simulink library "MOST_Lib"  (R2020b)
settings: Solver=VariableStepAuto  StartTime=0.0  StopTime=10.0  RelTol=1e-3
undefined symbols (not defined in the file or in its folder): simu, wind, windTurbine, windturbine, X, Y, Z
libraries: sm_lib x34, nesl_utility x28, $bdroot x6, simulink_extras x5, simulink x4, WECSim_Lib_Body_Elements x1
(part 2 of 2)

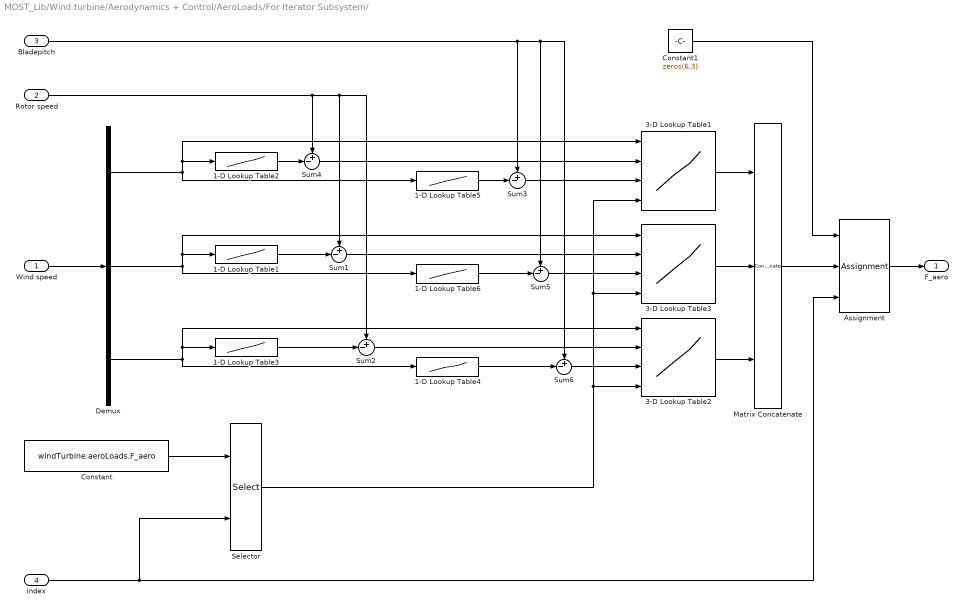
== system MOST_Lib/Wind turbine/Aerodynamics + Control/AeroLoads/For Iterator Subsystem/  ==
  Wind speed : Inport
  Rotor speed : Inport  Port=2
  Bladepitch : Inport  Port=3
  Index : Inport  Port=4
  1-D Lookup Table1 : Lookup_n-D  NumberOfTableDimensions=1  BreakpointsForDimension1=windTurbine.aeroLoads.SS.WINDSPEED  Table=windTurbine.aeroLoads.SS.ROTSPD  InternalRulePriority=Speed  RndMeth=Simplest  InputSameDT=on
  1-D Lookup Table2 : Lookup_n-D  NumberOfTableDimensions=1  BreakpointsForDimension1=windTurbine.aeroLoads.SS.WINDSPEED  Table=windTurbine.aeroLoads.SS.ROTSPD  InternalRulePriority=Speed  RndMeth=Simplest  InputSameDT=on
  1-D Lookup Table3 : Lookup_n-D  NumberOfTableDimensions=1  BreakpointsForDimension1=windTurbine.aeroLoads.SS.WINDSPEED  Table=windTurbine.aeroLoads.SS.ROTSPD  InternalRulePriority=Speed  RndMeth=Simplest  InputSameDT=on
  1-D Lookup Table4 : Lookup_n-D  NumberOfTableDimensions=1  BreakpointsForDimension1=windTurbine.aeroLoads.SS.WINDSPEED  Table=windTurbine.aeroLoads.SS.BLADEPITCH  InternalRulePriority=Speed  RndMeth=Simplest  InputSameDT=on
  1-D Lookup Table5 : Lookup_n-D  NumberOfTableDimensions=1  BreakpointsForDimension1=windTurbine.aeroLoads.SS.WINDSPEED  Table=windTurbine.aeroLoads.SS.BLADEPITCH  InternalRulePriority=Speed  RndMeth=Simplest  InputSameDT=on
  1-D Lookup Table6 : Lookup_n-D  NumberOfTableDimensions=1  BreakpointsForDimension1=windTurbine.aeroLoads.SS.WINDSPEED  Table=windTurbine.aeroLoads.SS.BLADEPITCH  InternalRulePriority=Speed  RndMeth=Simplest  InputSameDT=on
  3-D Lookup Table1 : Lookup_n-D  NumberOfTableDimensions=3  BreakpointsForDimension1=windTurbine.aeroLoads.V  BreakpointsForDimension2=windTurbine.aeroLoads.omegaerr  BreakpointsForDimension3=windTurbine.aeroLoads.thetaerr  TableSource=Input port  Table=squeeze(windturbine.aeroloads.F_aero(:,:,:,1))  InternalRulePriority=Speed  RndMeth=Simplest  InputSameDT=on
      settings: InputPortMap=u0,u1,u2,p221
  3-D Lookup Table2 : Lookup_n-D  NumberOfTableDimensions=3  BreakpointsForDimension1=windTurbine.aeroLoads.V  BreakpointsForDimension2=windTurbine.aeroLoads.omegaerr  BreakpointsForDimension3=windTurbine.aeroLoads.thetaerr  TableSource=Input port  Table=squeeze(windturbine.aeroloads.F_aero(:,:,:,1))  InternalRulePriority=Speed  RndMeth=Simplest  InputSameDT=on
      settings: InputPortMap=u0,u1,u2,p221
  3-D Lookup Table3 : Lookup_n-D  NumberOfTableDimensions=3  BreakpointsForDimension1=windTurbine.aeroLoads.V  BreakpointsForDimension2=windTurbine.aeroLoads.omegaerr  BreakpointsForDimension3=windTurbine.aeroLoads.thetaerr  TableSource=Input port  Table=squeeze(windturbine.aeroloads.F_aero(:,:,:,1))  InternalRulePriority=Speed  RndMeth=Simplest  InputSameDT=on
      settings: InputPortMap=u0,u1,u2,p221
  Assignment : Assignment  NumberOfDimensions=2  DiagnosticForDimensions=Warning  IndexOptions=Index vector (port),Assign all  Indices=1,1  OutputSizes=1,1
  Constant : Constant  Value=windTurbine.aeroLoads.F_aero
  Constant1 : Constant  Value=zeros(6,3)
  Demux : Demux  Outputs=3
  Matrix Concatenate : Concatenate  NumInputs=3  Mode=Multidimensional array  ConcatenateDimension=2
  Selector : Selector  NumberOfDimensions=4  InputPortWidth=3  IndexOptions=Select all,Select all,Select all,Index vector (port)  Indices=[1 3],1,1,1  OutputSizes=1,1,1,1
  Sum{1..6} (6 alike) : Sum  Inputs=+-|
  F_aero : Outport  PortDimensions=[6 3]
  wires:
  Wind speed/1 -> Demux/1
  Demux/3 -> 3-D Lookup Table2/1, 1-D Lookup Table3/1, 1-D Lookup Table4/1
  Demux/2 -> 1-D Lookup Table1/1, 3-D Lookup Table3/1, 1-D Lookup Table6/1
  1-D Lookup Table6/1 -> Sum5/2
  1-D Lookup Table5/1 -> Sum3/2
  1-D Lookup Table4/1 -> Sum6/2
  Sum6/1 -> 3-D Lookup Table2/3
  Sum4/1 -> 3-D Lookup Table1/2
  Sum3/1 -> 3-D Lookup Table1/3
  Demux/1 -> 1-D Lookup Table2/1, 3-D Lookup Table1/1, 1-D Lookup Table5/1
  Sum5/1 -> 3-D Lookup Table3/3
  Sum1/1 -> 3-D Lookup Table3/2
  Sum2/1 -> 3-D Lookup Table2/2
  Rotor speed/1 -> Sum4/1, Sum2/1, Sum1/1
  Constant/1 -> Selector/1
  Index/1 -> Selector/2, Assignment/3
  Selector/1 -> 3-D Lookup Table2/4, 3-D Lookup Table3/4, 3-D Lookup Table1/4
  1-D Lookup Table2/1 -> Sum4/2
  1-D Lookup Table1/1 -> Sum1/2
  1-D Lookup Table3/1 -> Sum2/2
  Bladepitch/1 -> Sum3/1, Sum6/1, Sum5/1
  Assignment/1 -> F_aero/1
  Constant1/1 -> Assignment/1
  3-D Lookup Table1/1 -> Matrix Concatenate/1
  3-D Lookup Table3/1 -> Matrix Concatenate/2
  3-D Lookup Table2/1 -> Matrix Concatenate/3
  Matrix Concatenate/1 -> Assignment/2

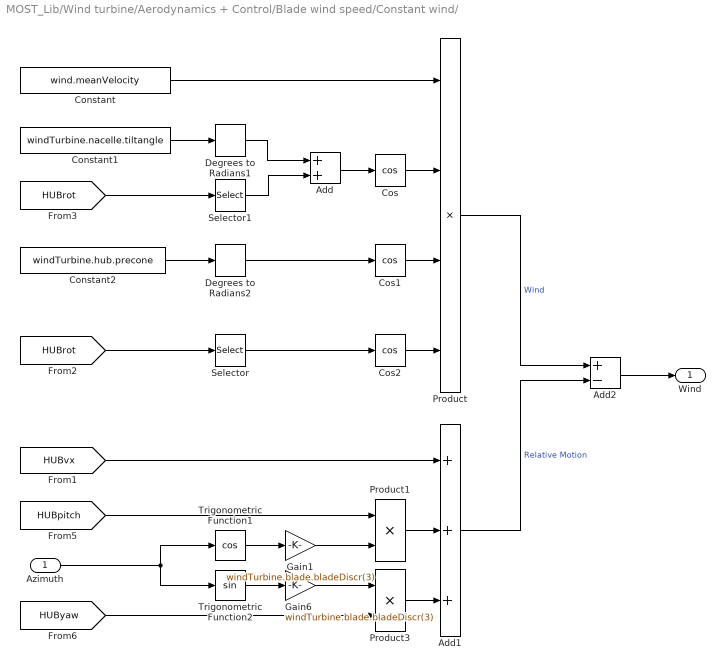
== system MOST_Lib/Wind turbine/Aerodynamics + Control/Blade wind speed/Constant wind/  ==
  Azimuth : Inport
  Add : Sum
  Add1 : Sum  Inputs=+++
  Add2 : Sum  Inputs=+-
  Constant : Constant  Value=wind.meanVelocity
  Constant1 : Constant  Value=windTurbine.nacelle.tiltangle
  Constant2 : Constant  Value=windTurbine.hub.precone
  Cos : Trigonometry  Operator=cos
  Cos1 : Trigonometry  Operator=cos
  Cos2 : Trigonometry  Operator=cos
  Degrees to Radians1 : simulink_extras/Transformations/Degrees to Radians
  Degrees to Radians2 : simulink_extras/Transformations/Degrees to Radians
  Gain1 : Gain  Gain=windTurbine.blade.bladeDiscr(3)
  Gain6 : Gain  Gain=windTurbine.blade.bladeDiscr(3)
  Product : Product  Inputs=4
  Product1 : Product
  Product3 : Product
  Selector : Selector  InputPortWidth=3  IndexOptions=Index vector (dialog)  Indices=[3]  OutputSizes=1
  Selector1 : Selector  InputPortWidth=3  IndexOptions=Index vector (dialog)  Indices=[2]  OutputSizes=1
  Trigonometric Function1 : Trigonometry  Operator=cos
  Trigonometric Function2 : Trigonometry
  Wind : Outport
  wires:
  Cos1/1 -> Product/3
  Selector/1 -> Cos2/1
  Degrees to Radians2/1 -> Cos1/1
  Add/1 -> Cos/1
  Selector1/1 -> Add/2
  Constant1/1 -> Degrees to Radians1/1
  Degrees to Radians1/1 -> Add/1
  Cos2/1 -> Product/4
  Constant2/1 -> Degrees to Radians2/1
  Cos/1 -> Product/2
  Product/1 -> Add2/1  "Wind"
  Constant/1 -> Product/1
  Product3/1 -> Add1/3
  Trigonometric Function2/1 -> Gain6/1
  Gain6/1 -> Product3/1
  Add1/1 -> Add2/2  "Relative Motion"
  [HUBvx] -> Add1/1
  Gain1/1 -> Product1/2
  Product1/1 -> Add1/2
  Azimuth/1 -> Trigonometric Function2/1, Trigonometric Function1/1
  Trigonometric Function1/1 -> Gain1/1
  Add2/1 -> Wind/1
  [HUBrot] -> Selector/1
  [HUBpitch] -> Product1/1
  [HUByaw] -> Product3/2
  [HUBrot] -> Selector1/1

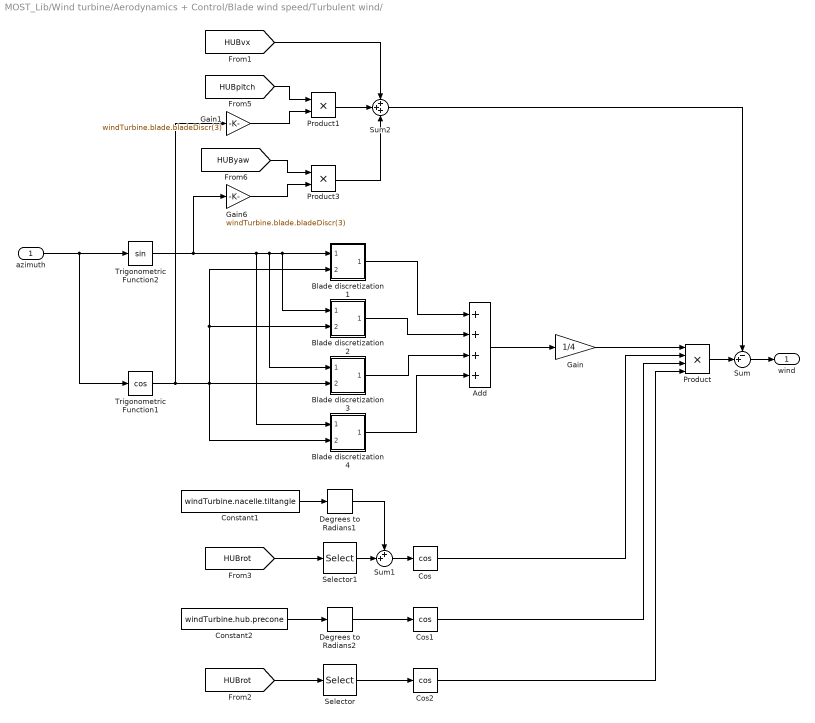
== system MOST_Lib/Wind turbine/Aerodynamics + Control/Blade wind speed/Turbulent wind/    ==
  azimuth : Inport
  Add : Sum  Inputs=++++
  Blade discretization 1 : Subsystem  mask{bladeDiscr=windTurbine.blade.bladeDiscr(1)}
  Blade discretization 2 : Subsystem  mask{bladeDiscr=windTurbine.blade.bladeDiscr(2)}
  Blade discretization 3 : Subsystem  mask{bladeDiscr=windTurbine.blade.bladeDiscr(3)}
  Blade discretization 4 : Subsystem  mask{bladeDiscr=windTurbine.blade.bladeDiscr(4)}
  Constant1 : Constant  Value=windTurbine.nacelle.tiltangle
  Constant2 : Constant  Value=windTurbine.hub.precone
  Cos : Trigonometry  Operator=cos
  Cos1 : Trigonometry  Operator=cos
  Cos2 : Trigonometry  Operator=cos
  Degrees to Radians1 : simulink_extras/Transformations/Degrees to Radians
  Degrees to Radians2 : simulink_extras/Transformations/Degrees to Radians
  Gain : Gain  Gain=1/4
  Gain1 : Gain  Gain=windTurbine.blade.bladeDiscr(3)
  Gain6 : Gain  Gain=windTurbine.blade.bladeDiscr(3)
  Product : Product  Inputs=4
  Product1 : Product
  Product3 : Product
  Selector : Selector  InputPortWidth=3  IndexOptions=Index vector (dialog)  Indices=[3]  OutputSizes=1
  Selector1 : Selector  InputPortWidth=3  IndexOptions=Index vector (dialog)  Indices=[2]  OutputSizes=1
  Sum : Sum  Inputs=-+|
  Sum1 : Sum  Inputs=++|
  Sum2 : Sum  Inputs=+++
  Trigonometric Function1 : Trigonometry  Operator=cos
  Trigonometric Function2 : Trigonometry
  wind : Outport
  wires:
  Gain/1 -> Product/1
  Cos2/1 -> Product/4
  Degrees to Radians1/1 -> Sum1/1
  Trigonometric Function1/1 -> Gain1/1, Blade discretization 1/2(In2), Blade discretization 2/2(In2), Blade discretization 3/2(In2), Blade discretization 4/2(In2)
  Blade discretization 2/1(Out1) -> Add/2
  Blade discretization 4/1(Out1) -> Add/4
  [HUBvx] -> Sum2/1
  Sum1/1 -> Cos/1
  Cos1/1 -> Product/3
  Product/1 -> Sum/2
  Constant1/1 -> Degrees to Radians1/1
  [HUBrot] -> Selector/1
  Product1/1 -> Sum2/2
  Selector/1 -> Cos2/1
  Degrees to Radians2/1 -> Cos1/1
  [HUBrot] -> Selector1/1
  Gain1/1 -> Product1/2
  Product3/1 -> Sum2/3
  Sum/1 -> wind/1
  Constant2/1 -> Degrees to Radians2/1
  Blade discretization 3/1(Out1) -> Add/3
  Blade discretization 1/1(Out1) -> Add/1
  azimuth/1 -> Trigonometric Function2/1, Trigonometric Function1/1
  Cos/1 -> Product/2
  Gain6/1 -> Product3/2
  Sum2/1 -> Sum/1
  [HUBpitch] -> Product1/1
  [HUByaw] -> Product3/1
  Trigonometric Function2/1 -> Gain6/1, Blade discretization 1/1(In1), Blade discretization 2/1(In1), Blade discretization 3/1(In1), Blade discretization 4/1(In1)
  Selector1/1 -> Sum1/2
  Add/1 -> Gain/1

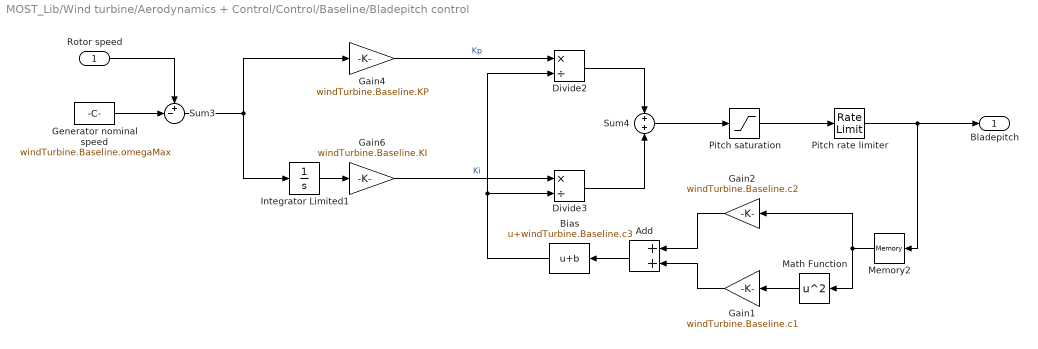
== system MOST_Lib/Wind turbine/Aerodynamics + Control/Control/Baseline/Bladepitch control ==
  Rotor speed : Inport
  Add : Sum
  Bias : Bias  Bias=windTurbine.Baseline.c3
  Divide2 : Product  Inputs=*/
  Divide3 : Product  Inputs=*/
  Gain1 : Gain  Gain=windTurbine.Baseline.c1
  Gain2 : Gain  Gain=windTurbine.Baseline.c2
  Gain4 : Gain  Gain=windTurbine.Baseline.KP
  Gain6 : Gain  Gain=windTurbine.Baseline.KI
  Generator nominal speed : Constant  Value=windTurbine.Baseline.omegaMax
  Integrator Limited1 : Integrator  LimitOutput=on  LowerSaturationLimit=0
  Math Function : Math  Operator=square  SaturateOnIntegerOverflow=on
  Memory2 : Memory
  Pitch rate limiter : RateLimiter  RisingSlewLimit=windTurbine.Baseline.thetaMaxRate  FallingSlewLimit=-windTurbine.Baseline.thetaMaxRate  SampleTimeMode=inherited
  Pitch saturation : Saturate  UpperLimit=pi/2  LowerLimit=0
  Sum3 : Sum  Inputs=+-|
  Sum4 : Sum
  Bladepitch : Outport  VectorParamsAs1DForOutWhenUnconnected=off
  wires:
  Integrator Limited1/1 -> Gain6/1
  Bias/1 -> Divide3/2, Divide2/2
  Add/1 -> Bias/1
  Sum4/1 -> Pitch saturation/1
  Generator nominal speed/1 -> Sum3/2
  Pitch rate limiter/1 -> Bladepitch/1, Memory2/1
  Pitch saturation/1 -> Pitch rate limiter/1
  Gain6/1 -> Divide3/1  "Ki"
  Sum3/1 -> Gain4/1, Integrator Limited1/1
  Gain4/1 -> Divide2/1  "Kp"
  Divide3/1 -> Sum4/2
  Divide2/1 -> Sum4/1
  Rotor speed/1 -> Sum3/1
  Gain1/1 -> Add/2
  Gain2/1 -> Add/1
  Math Function/1 -> Gain1/1
  Memory2/1 -> Math Function/1, Gain2/1

== system MOST_Lib/Wind turbine/Aerodynamics + Control/Control/ROSCO/ROSCO controller  ==
  Generator speed : Inport
  Wind speed : Inport  Port=2
  NacXdot : Inport  Port=3
  " " : StateSpace  A=windTurbine.ROSCO.omegaFilter.A  B=windTurbine.ROSCO.omegaFilter.B  C=windTurbine.ROSCO.omegaFilter.C  D=windTurbine.ROSCO.omegaFilter.D  InitialCondition=[0,(7.55*pi/30)/windTurbine.ROSCO.omegaFilter.C(2)]
  "  " : StateSpace  A=windTurbine.ROSCO.windFilter.A  B=windTurbine.ROSCO.windFilter.B  C=windTurbine.ROSCO.windFilter.C  D=windTurbine.ROSCO.windFilter.D  InitialCondition=[0,10/windTurbine.ROSCO.windFilter.C(2)]
  Bladepitch control : Subsystem
  Mux : Mux  Inputs=3
  Setpoint smoother : Subsystem
  Torque control : Subsystem
  Transfer Fcn : TransferFcn  Denominator=[windTurbine.ROSCO.pitchFilter.A windTurbine.ROSCO.pitchFilter.B 1]
  Gen torque : Outport
  Bladepitch : Outport  Port=2
  wires:
  "  "/1 -> Torque control/2(Wind speed), Bladepitch control/2(Wind speed)
  Mux/1 -> Bladepitch/1
  NacXdot/1 -> Transfer Fcn/1
  Bladepitch control/1(Bladepitch) -> Mux/3, Mux/1, Setpoint smoother/2(Bladepitch), Mux/2
  Torque control/1(Gen torque) -> Gen torque/1, Setpoint smoother/1(Gen torque)
  Transfer Fcn/1 -> Bladepitch control/4(NacXdot)
  " "/1 -> Bladepitch control/3(Gen speed), Torque control/1(Gen speed)
  Generator speed/1 -> " "/1
  Setpoint smoother/1(Del_Omega_g) -> Bladepitch control/1(Del_Omega_g), Torque control/3(Del_omega_g)
  Wind speed/1 -> "  "/1

== system MOST_Lib/Wind turbine/Aerodynamics + Control/Blade wind speed/Turbulent wind/   /Blade discretization 1 ==
  In1 : Inport
  In2 : Inport  Port=2
  Bias : Bias  Bias=windTurbine.hub.height
  Clock : Clock
  Wind : Lookup_n-D  NumberOfTableDimensions=3  BreakpointsForDimension1=wind.time  BreakpointsForDimension2=wind.yDiscr  BreakpointsForDimension3=wind.zDiscr  BeginIndexSearchUsingPreviousIndexResult=on  Table=wind.velocity  InternalRulePriority=Speed  RndMeth=Simplest  InputSameDT=on
      settings: InputPortMap=u0,u1,u2
  r : Gain  Gain=bladeDiscr
  r1 : Gain  Gain=bladeDiscr
  Out1 : Outport
  wires:
  r1/1 -> Bias/1
  r/1 -> Wind/2
  Clock/1 -> Wind/1
  Wind/1 -> Out1/1
  In2/1 -> r1/1
  Bias/1 -> Wind/3
  In1/1 -> r/1

== system MOST_Lib/Wind turbine/Aerodynamics + Control/Blade wind speed/Turbulent wind/   /Blade discretization 2 ==
  In1 : Inport
  In2 : Inport  Port=2
  Bias : Bias  Bias=windTurbine.hub.height
  Clock : Clock
  Wind : Lookup_n-D  NumberOfTableDimensions=3  BreakpointsForDimension1=wind.time  BreakpointsForDimension2=wind.yDiscr  BreakpointsForDimension3=wind.zDiscr  BeginIndexSearchUsingPreviousIndexResult=on  Table=wind.velocity  InternalRulePriority=Speed  RndMeth=Simplest  InputSameDT=on
      settings: InputPortMap=u0,u1,u2
  r : Gain  Gain=bladeDiscr
  r1 : Gain  Gain=bladeDiscr
  Out1 : Outport
  wires:
  In1/1 -> r/1
  Bias/1 -> Wind/3
  In2/1 -> r1/1
  Wind/1 -> Out1/1
  Clock/1 -> Wind/1
  r/1 -> Wind/2
  r1/1 -> Bias/1

== system MOST_Lib/Wind turbine/Aerodynamics + Control/Blade wind speed/Turbulent wind/   /Blade discretization 3 ==
  In1 : Inport
  In2 : Inport  Port=2
  Bias : Bias  Bias=windTurbine.hub.height
  Clock : Clock
  Wind : Lookup_n-D  NumberOfTableDimensions=3  BreakpointsForDimension1=wind.time  BreakpointsForDimension2=wind.yDiscr  BreakpointsForDimension3=wind.zDiscr  BeginIndexSearchUsingPreviousIndexResult=on  Table=wind.velocity  InternalRulePriority=Speed  RndMeth=Simplest  InputSameDT=on
      settings: InputPortMap=u0,u1,u2
  r : Gain  Gain=bladeDiscr
  r1 : Gain  Gain=bladeDiscr
  Out1 : Outport
  wires:
  r1/1 -> Bias/1
  r/1 -> Wind/2
  Clock/1 -> Wind/1
  Wind/1 -> Out1/1
  In2/1 -> r1/1
  Bias/1 -> Wind/3
  In1/1 -> r/1

== system MOST_Lib/Wind turbine/Aerodynamics + Control/Blade wind speed/Turbulent wind/   /Blade discretization 4 ==
  In1 : Inport
  In2 : Inport  Port=2
  Bias : Bias  Bias=windTurbine.hub.height
  Clock : Clock
  Wind : Lookup_n-D  NumberOfTableDimensions=3  BreakpointsForDimension1=wind.time  BreakpointsForDimension2=wind.yDiscr  BreakpointsForDimension3=wind.zDiscr  BeginIndexSearchUsingPreviousIndexResult=on  Table=wind.velocity  InternalRulePriority=Speed  RndMeth=Simplest  InputSameDT=on
      settings: InputPortMap=u0,u1,u2
  r : Gain  Gain=bladeDiscr
  r1 : Gain  Gain=bladeDiscr
  Out1 : Outport
  wires:
  In1/1 -> r/1
  Bias/1 -> Wind/3
  In2/1 -> r1/1
  Wind/1 -> Out1/1
  Clock/1 -> Wind/1
  r/1 -> Wind/2
  r1/1 -> Bias/1

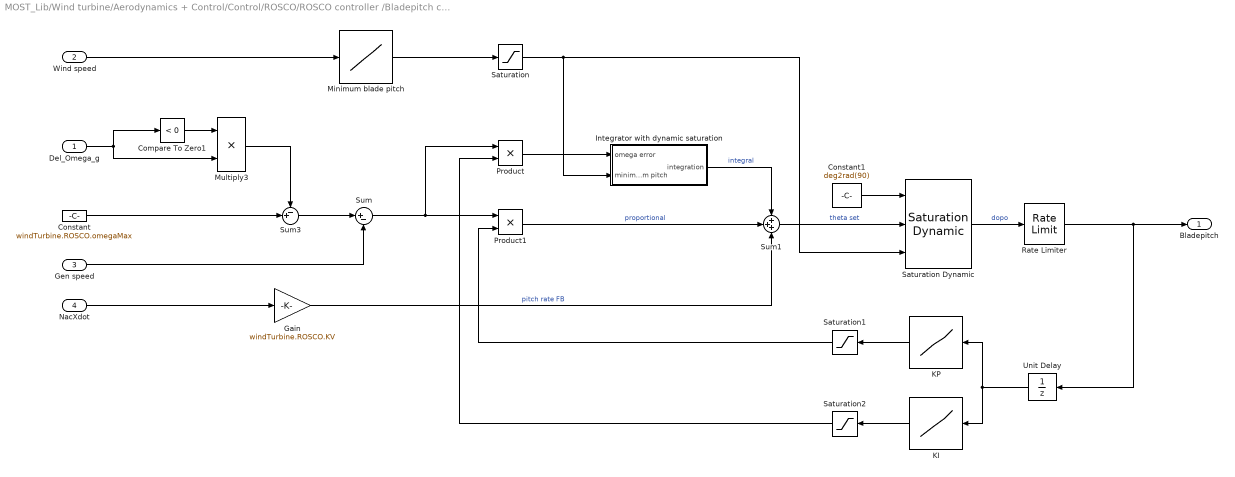
== system MOST_Lib/Wind turbine/Aerodynamics + Control/Control/ROSCO/ROSCO controller /Bladepitch control ==
  Del_Omega_g : Inport
  Wind speed : Inport  Port=2
  Gen speed : Inport  Port=3
  NacXdot : Inport  Port=4
  Compare To Zero1 : simulink/Logic and Bit Operations/Compare To Zero  relop=<  OutDataTypeStr=boolean
  Constant : Constant  Value=windTurbine.ROSCO.omegaMax
  Constant1 : Constant  Value=deg2rad(90)
  Gain : Gain  Gain=windTurbine.ROSCO.KV
  Integrator with dynamic saturation : Subsystem
  KI : Lookup_n-D  NumberOfTableDimensions=1  BreakpointsForDimension1=windTurbine.ROSCO.theta  Table=windTurbine.ROSCO.KI_theta  InternalRulePriority=Speed  RndMeth=Simplest  InputSameDT=on
  KP : Lookup_n-D  NumberOfTableDimensions=1  BreakpointsForDimension1=windTurbine.ROSCO.theta  Table=windTurbine.ROSCO.KP_theta  InternalRulePriority=Speed  RndMeth=Simplest  InputSameDT=on
  "Minimum blade pitch " : Lookup_n-D  NumberOfTableDimensions=1  BreakpointsForDimension1=windTurbine.ROSCO.WS  Table=windTurbine.ROSCO.MINBLADEPITCH  InternalRulePriority=Speed  RndMeth=Simplest  InputSameDT=on
  Multiply3 : Product
  Product : Product
  Product1 : Product
  "Rate Limiter " : RateLimiter  RisingSlewLimit=windTurbine.ROSCO.thetaMaxRate  FallingSlewLimit=-windTurbine.ROSCO.thetaMaxRate  SampleTimeMode=inherited
  Saturation : Saturate  UpperLimit=deg2rad(90)  LowerLimit=0
  "Saturation Dynamic " : simulink/Discontinuities/Saturation Dynamic  OutMin=[]  OutMax=[]  OutDataTypeStr=Inherit: Same as second input  OutDataType=fixdt(1, 16)  OutScaling=2^-10  DoSatur=off
  Saturation1 : Saturate  UpperLimit=windTurbine.ROSCO.KP_theta(end)  LowerLimit=windTurbine.ROSCO.KP_theta(1)
  Saturation2 : Saturate  UpperLimit=windTurbine.ROSCO.KI_theta(end)  LowerLimit=windTurbine.ROSCO.KI_theta(1)
  Sum : Sum  Inputs=|+-
  Sum1 : Sum  Inputs=+++
  Sum3 : Sum  Inputs=-+|
  Unit Delay : UnitDelay  SampleTime=-1  HasFrameUpgradeWarning=on
  Bladepitch : Outport
  wires:
  Saturation/1 -> "Saturation Dynamic "/3, Integrator with dynamic saturation/2(minimum pitch)
  "Rate Limiter "/1 -> Unit Delay/1, Bladepitch/1
  NacXdot/1 -> Gain/1
  "Saturation Dynamic "/1 -> "Rate Limiter "/1  "dopo"
  Sum3/1 -> Sum/1
  Constant/1 -> Sum3/2
  Del_Omega_g/1 -> Compare To Zero1/1, Multiply3/2
  Compare To Zero1/1 -> Multiply3/1
  Multiply3/1 -> Sum3/1
  KI/1 -> Saturation2/1
  KP/1 -> Saturation1/1
  Unit Delay/1 -> KI/1, KP/1
  Sum1/1 -> "Saturation Dynamic "/2  "theta set"
  Integrator with dynamic saturation/1(integration) -> Sum1/1  "integral"
  Product1/1 -> Sum1/2  "proportional"
  Sum/1 -> Product1/1, Product/1
  Product/1 -> Integrator with dynamic saturation/1(omega error)
  Constant1/1 -> "Saturation Dynamic "/1
  "Minimum blade pitch "/1 -> Saturation/1
  Wind speed/1 -> "Minimum blade pitch "/1
  Gen speed/1 -> Sum/2
  Saturation1/1 -> Product1/2
  Saturation2/1 -> Product/2
  Gain/1 -> Sum1/3  "pitch rate FB"

== system MOST_Lib/Wind turbine/Aerodynamics + Control/Control/ROSCO/ROSCO controller /Setpoint smoother ==
  Gen torque : Inport
  Bladepitch : Inport  Port=2
  Bias : Bias  Bias=-windTurbine.ROSCO.torqueMax
  Gain : Gain  Gain=windTurbine.ROSCO.SPS_kb/deg2rad(30)
  Gain1 : Gain  Gain=-windTurbine.ROSCO.SPS_kt/windTurbine.ROSCO.torqueMax
  Gain2 : Gain  Gain=windTurbine.ROSCO.omegaMax
  Sum2 : Sum  Inputs=+|-
  Transfer Fcn : TransferFcn  Denominator=[windTurbine.ROSCO.SPSFilter.A 1]
  Del_Omega_g : Outport  VectorParamsAs1DForOutWhenUnconnected=off
  wires:
  Gen torque/1 -> Bias/1
  Bladepitch/1 -> Gain/1
  Gain2/1 -> Del_Omega_g/1
  Bias/1 -> Gain1/1
  Sum2/1 -> Transfer Fcn/1
  Transfer Fcn/1 -> Gain2/1
  Gain/1 -> Sum2/1
  Gain1/1 -> Sum2/2

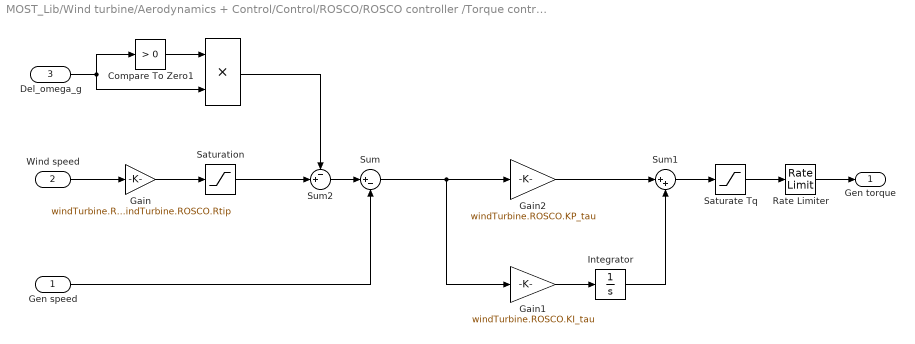
== system MOST_Lib/Wind turbine/Aerodynamics + Control/Control/ROSCO/ROSCO controller /Torque control ==
  Gen speed : Inport
  Wind speed : Inport  Port=2
  Del_omega_g : Inport  Port=3
  "  " : Product
  Compare To Zero1 : simulink/Logic and Bit Operations/Compare To Zero  relop=>  OutDataTypeStr=boolean
  Gain : Gain  Gain=windTurbine.ROSCO.TSR_opt/windTurbine.ROSCO.Rtip
  Gain1 : Gain  Gain=windTurbine.ROSCO.KI_tau
  Gain2 : Gain  Gain=windTurbine.ROSCO.KP_tau
  Integrator : Integrator  LimitOutput=on  UpperSaturationLimit=windTurbine.ROSCO.torqueMax  LowerSaturationLimit=0
  "Rate Limiter " : RateLimiter  RisingSlewLimit=windTurbine.ROSCO.torqueMaxRate  FallingSlewLimit=-windTurbine.ROSCO.torqueMaxRate  SampleTimeMode=inherited
  Saturate Tq : Saturate  UpperLimit=windTurbine.ROSCO.torqueMax  LowerLimit=0
  Saturation : Saturate  UpperLimit=windTurbine.ROSCO.omegaMax  LowerLimit=windTurbine.ROSCO.omegaMin
  Sum : Sum  Inputs=|+-
  Sum1 : Sum  Inputs=|++
  Sum2 : Sum  Inputs=-+|
  Gen torque : Outport
  wires:
  Integrator/1 -> Sum1/2
  Gain1/1 -> Integrator/1
  Gain2/1 -> Sum1/1
  Saturate Tq/1 -> "Rate Limiter "/1
  "Rate Limiter "/1 -> Gen torque/1
  Sum2/1 -> Sum/1
  Del_omega_g/1 -> Compare To Zero1/1, "  "/2
  Compare To Zero1/1 -> "  "/1
  "  "/1 -> Sum2/1
  Sum/1 -> Gain2/1, Gain1/1
  Saturation/1 -> Sum2/2
  Gain/1 -> Saturation/1
  Sum1/1 -> Saturate Tq/1
  Gen speed/1 -> Sum/2
  Wind speed/1 -> Gain/1

== system MOST_Lib/Wind turbine/Aerodynamics + Control/Control/ROSCO/ROSCO controller /Bladepitch control/Integrator with dynamic saturation ==
  omega error : Inport
  minimum pitch : Inport  Port=2
  Constant : Constant  Value=deg2rad(90)
  Gain : Gain  Gain=simu.dt
  Saturation Dynamic : simulink/Discontinuities/Saturation Dynamic  OutMin=[]  OutMax=[]  OutDataTypeStr=Inherit: Same as second input  OutDataType=fixdt(1, 16)  OutScaling=2^-10  DoSatur=off
  Sum : Sum  Inputs=|++
  Unit Delay : UnitDelay  SampleTime=-1  HasFrameUpgradeWarning=on
  integration : Outport
  wires:
  minimum pitch/1 -> Saturation Dynamic/3
  Gain/1 -> Sum/1
  Unit Delay/1 -> Sum/2
  Sum/1 -> Saturation Dynamic/2
  omega error/1 -> Gain/1
  Constant/1 -> Saturation Dynamic/1
  Saturation Dynamic/1 -> Unit Delay/1, integration/1
